simulink model "rfi_blk_v6_casper"  (Simulink 7.1)
settings: Solver=ode45  StartTime=0.0  StopTime=10.0  RelTol=1e-3
undefined symbols (not defined in the file): a, b
libraries: xbsIndex_r4 x13084, xps_library x4
(part 1 of 51)
settings Model: Creator=kaushal  LastModifiedBy=kaushal

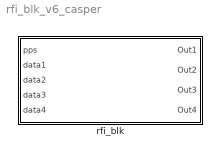
== system rfi_blk_v6_casper ==
  rfi_blk : Subsystem

== system rfi_blk_v6_casper/rfi_blk ==
  note: Median Absolute Deviation(MAD)
      |  Compuation Block
      | Computes median and MAD on 1024 bytes
      | of data for contiguous windows
      | Uses 1pps signal (active high - 1 clk) to restart
      | the computation
  note: RFI detection and filtering block
  note: RFI detection and filtering block
  note: RFI detection and filtering block
  note: RFI detection and filtering block
  note: 'n' value in median+/-n*sigma
  note: 0: Bypass
      | 1: Replace by constant value
      | 2: Replace by median value
      | 3: Replace with Digital Noise
  note: This design is divided into two blocks - MAD computation block and RFI detection and Filtering blocks
      | In the current version four RFI detection and filtering blocks are provided to cater to the four lanes from the ADC
  note: Replacement value for option 1 of bypass_mux
  pps : Inport  Orientation=up
  data1 : Inport  Port=2
  data2 : Inport  Port=3
  data3 : Inport  Port=4
  data4 : Inport  Port=5
  Constant1 : Constant  Value=10  OutDataType=sfix(16)  OutScaling=2^0
  Constant2 : Constant  Value=0  OutDataType=sfix(16)  OutScaling=2^0
  Constant3 : Constant  Value=3  OutDataType=sfix(16)  OutScaling=2^0
  Register{1,7..9} (4 alike) : xbsIndex_r4/Register  init=0  rst=off  en=off  dbl_ovrd=off  block_type=register
      settings: block_version=11.4
  Register{2..4,6} (4 alike) : xbsIndex_r4/Register  Orientation=up  init=0  rst=off  en=off  dbl_ovrd=off  block_type=register
      settings: block_version=11.4
  Reinterpret{9..12} (4 alike) : xbsIndex_r4/Reinterpret  force_arith_type=on  arith_type="Signed  (2's comp)"  force_bin_pt=on  bin_pt=0  block_type=reinterpret
      settings: infoedit="Changes signal type without altering the binary representation.   You can changed the signal between signed and unsigned, and relocate the binary point.<br><br>Hardware notes: In hardware this block costs nothing.<br><br>Example:  Suppose the input is 6 bits wide, signed, with 2 fractional bits, and the output is forced to unsigned with 0 fractional bits.  Then an input of -2.0 (1110.00 in binary 2's complement) becomes an output of 56 (111000 in binary)."
  Slice : xbsIndex_r4/Slice  nbits=8  boolean_output=off  mode=Lower Bit Location + Width  bit1=0  base1=MSB of Input  bit0=0  base0=LSB of Input  dbl_ovrd=off  block_type=slice
  Slice1 : xbsIndex_r4/Slice  nbits=8  boolean_output=off  mode=Lower Bit Location + Width  bit1=0  base1=MSB of Input  bit0=0  base0=LSB of Input  dbl_ovrd=off  block_type=slice
  Slice2 : xbsIndex_r4/Slice  nbits=2  boolean_output=off  mode=Lower Bit Location + Width  bit1=0  base1=MSB of Input  bit0=0  base0=LSB of Input  dbl_ovrd=off  block_type=slice
  bypass_mux : xps_library/software register  Tag=xps:sw_reg  SystemSampleTime=-1  io_dir=From Processor  arith_type=Unsigned  bitwidth=32  bin_pt=0  sample_period=1  gw_name=two_adc_rfi_v6_rfi_blk_bypass_mux_user_data_out
  cr1 : Subsystem
  cr2 : Subsystem
  cr4 : Subsystem
  cr5 : Subsystem
  mad_comp : Subsystem
  replacement : xps_library/software register  Tag=xps:sw_reg  SystemSampleTime=-1  io_dir=From Processor  arith_type=Unsigned  bitwidth=32  bin_pt=0  sample_period=1  gw_name=two_adc_rfi_v6_rfi_blk_replacement_user_data_out
  thres_fact : xps_library/software register  Tag=xps:sw_reg  SystemSampleTime=-1  io_dir=From Processor  arith_type=Unsigned  bitwidth=32  bin_pt=0  sample_period=1  gw_name=two_adc_rfi_v6_rfi_blk_thres_fact_user_data_out
  Out1 : Outport
  Out2 : Outport  Port=2
  Out3 : Outport  Port=3
  Out4 : Outport  Port=4
  wires:
  cr1/1(filter_o) -> Register1/1
  data2/1 -> Register7/1
  [med_valid] -> cr4/4(med_valid)
  [mad_valid] -> cr4/3(mad_valid)
  [mad] -> cr4/2(mad)
  [median] -> cr4/1(median)
  mad_comp/1(med_valid) -> [med_valid]
  mad_comp/2(median) -> [median]
  mad_comp/3(mad_valid) -> [mad_valid]
  mad_comp/4(mad) -> [mad]
  [med_valid] -> cr1/4(med_valid)
  [mad_valid] -> cr1/3(mad_valid)
  [mad] -> cr1/2(mad)
  [median] -> cr1/1(median)
  cr4/1(filter_o) -> Register2/1
  data3/1 -> Register8/1
  [med_valid] -> cr2/4(med_valid)
  [mad_valid] -> cr2/3(mad_valid)
  [mad] -> cr2/2(mad)
  [median] -> cr2/1(median)
  cr2/1(filter_o) -> Register3/1
  data4/1 -> Register9/1
  [med_valid] -> cr5/4(med_valid)
  [mad_valid] -> cr5/3(mad_valid)
  [mad] -> cr5/2(mad)
  [median] -> cr5/1(median)
  cr5/1(filter_o) -> Register4/1
  Reinterpret9/1 -> cr1/5(data_i)
  Reinterpret10/1 -> cr4/5(data_i)
  Reinterpret11/1 -> cr2/5(data_i)
  Reinterpret12/1 -> cr5/5(data_i)
  Register1/1 -> Out1/1
  Register2/1 -> Out2/1
  Register3/1 -> Out3/1
  Register4/1 -> Out4/1
  Register7/1 -> Reinterpret10/1
  Register8/1 -> Reinterpret11/1
  Register9/1 -> Reinterpret12/1
  pps/1 -> mad_comp/1(pps)
  data1/1 -> Register6/1
  thres_fact/1 -> Slice/1
  Constant1/1 -> thres_fact/1
  replacement/1 -> Slice1/1
  Constant2/1 -> replacement/1
  bypass_mux/1 -> Slice2/1
  Constant3/1 -> bypass_mux/1
  [thres] -> cr1/6(thr)
  [rep] -> cr1/7(rep)
  [bymux] -> cr1/8(bymux)
  Slice/1 -> [thres]
  Slice1/1 -> [rep]
  Slice2/1 -> [bymux]
  [thres] -> cr4/6(thr)
  [rep] -> cr4/7(rep)
  [bymux] -> cr4/8(bymux)
  [thres] -> cr2/6(thr)
  [rep] -> cr2/7(rep)
  [bymux] -> cr2/8(bymux)
  [thres] -> cr5/6(thr)
  [rep] -> cr5/7(rep)
  [bymux] -> cr5/8(bymux)
  Register6/1 -> mad_comp/2(data), Reinterpret9/1

== system rfi_blk_v6_casper/rfi_blk/cr1 ==
  note: Detection and Filtering (Clipping and Replacement) block
  median : Inport
  mad : Inport  Port=2
  mad_valid : Inport  Port=3
  med_valid : Inport  Port=4
  data_i : Inport  Port=5
  thr : Inport  Port=6
  rep : Inport  Port=7
  bymux : Inport  Port=8
  cr : Subsystem
  filter_o : Outport
  detect_o : Outport  Port=2
  data_o : Outport  Port=3
  wires:
  median/1 -> cr/1(median)
  cr/1(filter_o) -> filter_o/1
  mad/1 -> cr/2(mad1)
  mad_valid/1 -> cr/3(mad_valid)
  med_valid/1 -> cr/4(med_valid)
  cr/2(detect_o) -> detect_o/1
  data_i/1 -> cr/5(data_i)
  cr/3(data_o) -> data_o/1
  thr/1 -> cr/6(thres_fact)
  rep/1 -> cr/7(repl_val)
  bymux/1 -> cr/8(byp_mux)

== system rfi_blk_v6_casper/rfi_blk/cr2 ==
  note: Detection and Filtering (Clipping and Replacement) block
  median : Inport
  mad : Inport  Port=2
  mad_valid : Inport  Port=3
  med_valid : Inport  Port=4
  data_i : Inport  Port=5
  thr : Inport  Port=6
  rep : Inport  Port=7
  bymux : Inport  Port=8
  cr : Subsystem
  filter_o : Outport
  detect_o : Outport  Port=2
  data_o : Outport  Port=3
  wires:
  median/1 -> cr/1(median)
  cr/1(filter_o) -> filter_o/1
  mad/1 -> cr/2(mad1)
  mad_valid/1 -> cr/3(mad_valid)
  med_valid/1 -> cr/4(med_valid)
  cr/2(detect_o) -> detect_o/1
  data_i/1 -> cr/5(data_i)
  cr/3(data_o) -> data_o/1
  thr/1 -> cr/6(thres_fact)
  rep/1 -> cr/7(repl_val)
  bymux/1 -> cr/8(byp_mux)

== system rfi_blk_v6_casper/rfi_blk/cr4 ==
  note: Detection and Filtering (Clipping and Replacement) block
  median : Inport
  mad : Inport  Port=2
  mad_valid : Inport  Port=3
  med_valid : Inport  Port=4
  data_i : Inport  Port=5
  thr : Inport  Port=6
  rep : Inport  Port=7
  bymux : Inport  Port=8
  cr : Subsystem
  filter_o : Outport
  detect_o : Outport  Port=2
  data_o : Outport  Port=3
  wires:
  median/1 -> cr/1(median)
  cr/1(filter_o) -> filter_o/1
  mad/1 -> cr/2(mad1)
  mad_valid/1 -> cr/3(mad_valid)
  med_valid/1 -> cr/4(med_valid)
  cr/2(detect_o) -> detect_o/1
  data_i/1 -> cr/5(data_i)
  cr/3(data_o) -> data_o/1
  thr/1 -> cr/6(thres_fact)
  rep/1 -> cr/7(repl_val)
  bymux/1 -> cr/8(byp_mux)

== system rfi_blk_v6_casper/rfi_blk/cr5 ==
  note: Detection and Filtering (Clipping and Replacement) block
  median : Inport
  mad : Inport  Port=2
  mad_valid : Inport  Port=3
  med_valid : Inport  Port=4
  data_i : Inport  Port=5
  thr : Inport  Port=6
  rep : Inport  Port=7
  bymux : Inport  Port=8
  cr : Subsystem
  filter_o : Outport
  detect_o : Outport  Port=2
  data_o : Outport  Port=3
  wires:
  median/1 -> cr/1(median)
  cr/1(filter_o) -> filter_o/1
  mad/1 -> cr/2(mad1)
  mad_valid/1 -> cr/3(mad_valid)
  med_valid/1 -> cr/4(med_valid)
  cr/2(detect_o) -> detect_o/1
  data_i/1 -> cr/5(data_i)
  cr/3(data_o) -> data_o/1
  thr/1 -> cr/6(thres_fact)
  rep/1 -> cr/7(repl_val)
  bymux/1 -> cr/8(byp_mux)

== system rfi_blk_v6_casper/rfi_blk/mad_comp ==
  note: 0: Select pre-stored values from memory
      | 1: Input data
  note: First Median Computation
  note: Subtraction and Absolute Value Conversion
  note: Second Median Computation
  pps : Inport
  data : Inport  Port=2
  Constant1 : Constant  Value=0  OutDataType=sfix(16)  OutScaling=2^0
  Counter1 : xbsIndex_r4/Counter  cnt_type=Free Running  cnt_to=2048  operation=Up  start_count=0  cnt_by_val=1  arith_type=Unsigned  n_bits=13  bin_pt=0  load_pin=off  rst=off  en=off  explicit_period=on  period=1  dbl_ovrd=off  use_behavioral_HDL=off  implementation=Fabric  use_rpm=off  block_type=counter
  Delay2 : xbsIndex_r4/Delay  en=off  latency=3  dbl_ovrd=off  reg_retiming=off  block_type=delay
  Delay3 : xbsIndex_r4/Delay  Orientation=down  en=off  latency=1027+3  dbl_ovrd=off  reg_retiming=off  block_type=delay
  Delay4 : xbsIndex_r4/Delay  Orientation=down  en=off  latency=1027  dbl_ovrd=off  reg_retiming=off  block_type=delay
  Median1 : Subsystem
  Median2 : Subsystem
  Mux2 : xbsIndex_r4/Mux  inputs=2  en=off  latency=0  precision=User Defined  arith_type="Signed  (2's comp)"  n_bits=8  bin_pt=0  quantization=Truncate  overflow=Wrap  dbl_ovrd=off  block_type=mux
  ROM : xbsIndex_r4/ROM  depth=8192  initVector=0  distributed_mem=Block RAM  rst=off  init_reg=0  en=off  latency=1  arith_type="Signed  (2's comp)"  n_bits=8  bin_pt=0  dbl_ovrd=off  optimize=Area  use_rpm=on  block_type=sprom
      settings: block_version=10.1.3
  Reinterpret9 : xbsIndex_r4/Reinterpret  force_arith_type=on  arith_type="Signed  (2's comp)"  force_bin_pt=on  bin_pt=0  block_type=reinterpret
      settings: infoedit="Changes signal type without altering the binary representation.   You can changed the signal between signed and unsigned, and relocate the binary point.<br><br>Hardware notes: In hardware this block costs nothing.<br><br>Example:  Suppose the input is 6 bits wide, signed, with 2 fractional bits, and the output is forced to unsigned with 0 fractional bits.  Then an input of -2.0 (1110.00 in binary 2's complement) becomes an output of 56 (111000 in binary)."
  Scope3 : Scope  NumInputPorts=5
      settings: YMin=-1~5.7~-1~-1~-1  YMax=1~6.3~1~1~1  SaveName=ScopeData19  MaxDataPoints=30000
  Scope4 : Scope  NumInputPorts=5
      settings: YMin=81.7~-1~0.95~0.95~-1  YMax=90.3~1~1.05~1.05~1  SaveName=ScopeData22  MaxDataPoints=30000
  Slice : xbsIndex_r4/Slice  nbits=8  boolean_output=on  mode=Lower Bit Location + Width  bit1=0  base1=MSB of Input  bit0=0  base0=LSB of Input  dbl_ovrd=off  block_type=slice
  block_ram : Subsystem
  input_mux : xps_library/software register  Tag=xps:sw_reg  SystemSampleTime=-1  io_dir=From Processor  arith_type=Unsigned  bitwidth=32  bin_pt=0  sample_period=1  gw_name=two_adc_rfi_v6_rfi_blk_mad_comp_input_mux_user_data_out
  sub_abs_conv : Subsystem
  sync_control : Subsystem
  med_valid : Outport
  median : Outport  Port=2
  mad_valid : Outport  Port=3
  mad : Outport  Port=4
  wires:
  Counter1/1 -> ROM/1
  Median2/1(med_out) -> Delay4/1, sub_abs_conv/2(In2)
  sub_abs_conv/1(Out1) -> Median1/1(data_in)
  Median1/1(med_out) -> Scope4/1, mad/1
  Delay2/1 -> Median1/2(acc_reset), Scope4/3
  sync_control/1(Out1) -> Median2/2(acc_reset), Delay2/1
  block_ram/2(Out2) -> sub_abs_conv/1(In1)
  Median2/3(med_valid1) -> Delay3/1
  Median1/4(mad_valid) -> Scope4/4, mad_valid/1
  Median1/2(med_valid) -> Scope4/5
  Delay4/1 -> Scope3/2, median/1
  Delay3/1 -> Scope3/1, med_valid/1
  pps/1 -> sync_control/1(pps)
  Mux2/1 -> Median2/1(data_in), block_ram/1(data)
  data/1 -> Reinterpret9/1
  ROM/1 -> Mux2/3
  input_mux/1 -> Slice/1
  Constant1/1 -> input_mux/1
  Slice/1 -> Mux2/1
  Reinterpret9/1 -> Mux2/2

== system rfi_blk_v6_casper/rfi_blk/cr1/cr ==
  note: Detection Threshold Computation
  note: RFI Filtering (Clipping / Replacement)
  median : Inport
  mad1 : Inport  Port=2
  mad_valid : Inport  Port=3
  med_valid : Inport  Port=4
  data_i : Inport  Port=5
  thres_fact : Inport  Port=6
  repl_val : Inport  Port=7
  byp_mux : Inport  Port=8
  Delay1 : xbsIndex_r4/Delay  en=off  latency=10  dbl_ovrd=off  reg_retiming=off  block_type=delay
  Delay5 : xbsIndex_r4/Delay  en=off  latency=1027+1024  dbl_ovrd=off  reg_retiming=off  block_type=delay
  Mux1 : xbsIndex_r4/Mux  inputs=2  en=off  latency=0  precision=User Defined  arith_type="Signed  (2's comp)"  n_bits=8  bin_pt=0  quantization=Truncate  overflow=Wrap  dbl_ovrd=off  block_type=mux
  Mux2 : xbsIndex_r4/Mux  inputs=2  en=off  latency=0  precision=User Defined  arith_type=Unsigned  n_bits=8  bin_pt=0  quantization=Truncate  overflow=Wrap  dbl_ovrd=off  block_type=mux
  Register : xbsIndex_r4/Register  init=0  rst=off  en=off  dbl_ovrd=off  block_type=register
      settings: block_version=11.4
  Register1 : xbsIndex_r4/Register  init=0  rst=off  en=off  dbl_ovrd=off  block_type=register
      settings: block_version=11.4
  Scope1 : Scope  NumInputPorts=4
      settings: YMin=-1~-1~-150~-1  YMax=1~1~150~1  MaxDataPoints=30000
  Scope2 : Scope  NumInputPorts=4
      settings: YMin=-1~0.95~-125~-1  YMax=1~1.05~150~1  MaxDataPoints=30000
  clip_repl : Subsystem
  thres_calc : Subsystem
  filter_o : Outport
  detect_o : Outport  Port=2
  data_o : Outport  Port=3
  wires:
  clip_repl/1(filter_o) -> Scope2/1, filter_o/1
  Delay5/1 -> Delay1/1
  clip_repl/3(input_data) -> data_o/1, Scope2/3
  clip_repl/2(detect_o) -> Scope2/2, detect_o/1
  thres_calc/3(sigma_mad_o) -> clip_repl/5(sigma_mad)
  Register1/1 -> thres_calc/1(mad), Mux2/2
  Mux2/1 -> Register1/1
  Register/1 -> Mux1/2, thres_calc/2(median), clip_repl/4(median)
  Mux1/1 -> Register/1
  Delay1/1 -> clip_repl/3(input), Scope1/3
  thres_calc/2(n_th) -> clip_repl/2(n_thres), Scope1/2
  thres_calc/1(p_th) -> clip_repl/1(p_thres), Scope1/1
  mad1/1 -> Mux2/3
  mad_valid/1 -> Mux2/1
  median/1 -> Mux1/3
  med_valid/1 -> Mux1/1
  data_i/1 -> Delay5/1
  thres_fact/1 -> thres_calc/3(thres_fact)
  repl_val/1 -> clip_repl/6(repl_val)
  byp_mux/1 -> clip_repl/7(bypass_mux)

== system rfi_blk_v6_casper/rfi_blk/cr2/cr ==
  note: Detection Threshold Computation
  note: RFI Filtering (Clipping / Replacement)
  median : Inport
  mad1 : Inport  Port=2
  mad_valid : Inport  Port=3
  med_valid : Inport  Port=4
  data_i : Inport  Port=5
  thres_fact : Inport  Port=6
  repl_val : Inport  Port=7
  byp_mux : Inport  Port=8
  Delay1 : xbsIndex_r4/Delay  en=off  latency=10  dbl_ovrd=off  reg_retiming=off  block_type=delay
  Delay5 : xbsIndex_r4/Delay  en=off  latency=1027+1024  dbl_ovrd=off  reg_retiming=off  block_type=delay
  Mux1 : xbsIndex_r4/Mux  inputs=2  en=off  latency=0  precision=User Defined  arith_type="Signed  (2's comp)"  n_bits=8  bin_pt=0  quantization=Truncate  overflow=Wrap  dbl_ovrd=off  block_type=mux
  Mux2 : xbsIndex_r4/Mux  inputs=2  en=off  latency=0  precision=User Defined  arith_type=Unsigned  n_bits=8  bin_pt=0  quantization=Truncate  overflow=Wrap  dbl_ovrd=off  block_type=mux
  Register : xbsIndex_r4/Register  init=0  rst=off  en=off  dbl_ovrd=off  block_type=register
      settings: block_version=11.4
  Register1 : xbsIndex_r4/Register  init=0  rst=off  en=off  dbl_ovrd=off  block_type=register
      settings: block_version=11.4
  Scope1 : Scope  NumInputPorts=4
      settings: YMin=-1~-1~-150~-1  YMax=1~1~150~1  MaxDataPoints=30000
  Scope2 : Scope  NumInputPorts=4
      settings: YMin=-1~0.95~-125~-1  YMax=1~1.05~150~1  MaxDataPoints=30000
  clip_repl : Subsystem
  thres_calc : Subsystem
  filter_o : Outport
  detect_o : Outport  Port=2
  data_o : Outport  Port=3
  wires:
  clip_repl/1(filter_o) -> Scope2/1, filter_o/1
  Delay5/1 -> Delay1/1
  clip_repl/3(input_data) -> data_o/1, Scope2/3
  clip_repl/2(detect_o) -> Scope2/2, detect_o/1
  thres_calc/3(sigma_mad_o) -> clip_repl/5(sigma_mad)
  Register1/1 -> thres_calc/1(mad), Mux2/2
  Mux2/1 -> Register1/1
  Register/1 -> Mux1/2, thres_calc/2(median), clip_repl/4(median)
  Mux1/1 -> Register/1
  Delay1/1 -> clip_repl/3(input), Scope1/3
  thres_calc/2(n_th) -> clip_repl/2(n_thres), Scope1/2
  thres_calc/1(p_th) -> clip_repl/1(p_thres), Scope1/1
  mad1/1 -> Mux2/3
  mad_valid/1 -> Mux2/1
  median/1 -> Mux1/3
  med_valid/1 -> Mux1/1
  data_i/1 -> Delay5/1
  thres_fact/1 -> thres_calc/3(thres_fact)
  repl_val/1 -> clip_repl/6(repl_val)
  byp_mux/1 -> clip_repl/7(bypass_mux)

== system rfi_blk_v6_casper/rfi_blk/cr4/cr ==
  note: Detection Threshold Computation
  note: RFI Filtering (Clipping / Replacement)
  median : Inport
  mad1 : Inport  Port=2
  mad_valid : Inport  Port=3
  med_valid : Inport  Port=4
  data_i : Inport  Port=5
  thres_fact : Inport  Port=6
  repl_val : Inport  Port=7
  byp_mux : Inport  Port=8
  Delay1 : xbsIndex_r4/Delay  en=off  latency=10  dbl_ovrd=off  reg_retiming=off  block_type=delay
  Delay5 : xbsIndex_r4/Delay  en=off  latency=1027+1024  dbl_ovrd=off  reg_retiming=off  block_type=delay
  Mux1 : xbsIndex_r4/Mux  inputs=2  en=off  latency=0  precision=User Defined  arith_type="Signed  (2's comp)"  n_bits=8  bin_pt=0  quantization=Truncate  overflow=Wrap  dbl_ovrd=off  block_type=mux
  Mux2 : xbsIndex_r4/Mux  inputs=2  en=off  latency=0  precision=User Defined  arith_type=Unsigned  n_bits=8  bin_pt=0  quantization=Truncate  overflow=Wrap  dbl_ovrd=off  block_type=mux
  Register : xbsIndex_r4/Register  init=0  rst=off  en=off  dbl_ovrd=off  block_type=register
      settings: block_version=11.4
  Register1 : xbsIndex_r4/Register  init=0  rst=off  en=off  dbl_ovrd=off  block_type=register
      settings: block_version=11.4
  Scope1 : Scope  NumInputPorts=4
      settings: YMin=-1~-1~-150~-1  YMax=1~1~150~1  MaxDataPoints=30000
  Scope2 : Scope  NumInputPorts=4
      settings: YMin=-1~0.95~-125~-1  YMax=1~1.05~150~1  MaxDataPoints=30000
  clip_repl : Subsystem
  thres_calc : Subsystem
  filter_o : Outport
  detect_o : Outport  Port=2
  data_o : Outport  Port=3
  wires:
  clip_repl/1(filter_o) -> Scope2/1, filter_o/1
  Delay5/1 -> Delay1/1
  clip_repl/3(input_data) -> data_o/1, Scope2/3
  clip_repl/2(detect_o) -> Scope2/2, detect_o/1
  thres_calc/3(sigma_mad_o) -> clip_repl/5(sigma_mad)
  Register1/1 -> thres_calc/1(mad), Mux2/2
  Mux2/1 -> Register1/1
  Register/1 -> Mux1/2, thres_calc/2(median), clip_repl/4(median)
  Mux1/1 -> Register/1
  Delay1/1 -> clip_repl/3(input), Scope1/3
  thres_calc/2(n_th) -> clip_repl/2(n_thres), Scope1/2
  thres_calc/1(p_th) -> clip_repl/1(p_thres), Scope1/1
  mad1/1 -> Mux2/3
  mad_valid/1 -> Mux2/1
  median/1 -> Mux1/3
  med_valid/1 -> Mux1/1
  data_i/1 -> Delay5/1
  thres_fact/1 -> thres_calc/3(thres_fact)
  repl_val/1 -> clip_repl/6(repl_val)
  byp_mux/1 -> clip_repl/7(bypass_mux)

== system rfi_blk_v6_casper/rfi_blk/cr5/cr ==
  note: Detection Threshold Computation
  note: RFI Filtering (Clipping / Replacement)
  median : Inport
  mad1 : Inport  Port=2
  mad_valid : Inport  Port=3
  med_valid : Inport  Port=4
  data_i : Inport  Port=5
  thres_fact : Inport  Port=6
  repl_val : Inport  Port=7
  byp_mux : Inport  Port=8
  Delay1 : xbsIndex_r4/Delay  en=off  latency=10  dbl_ovrd=off  reg_retiming=off  block_type=delay
  Delay5 : xbsIndex_r4/Delay  en=off  latency=1027+1024  dbl_ovrd=off  reg_retiming=off  block_type=delay
  Mux1 : xbsIndex_r4/Mux  inputs=2  en=off  latency=0  precision=User Defined  arith_type="Signed  (2's comp)"  n_bits=8  bin_pt=0  quantization=Truncate  overflow=Wrap  dbl_ovrd=off  block_type=mux
  Mux2 : xbsIndex_r4/Mux  inputs=2  en=off  latency=0  precision=User Defined  arith_type=Unsigned  n_bits=8  bin_pt=0  quantization=Truncate  overflow=Wrap  dbl_ovrd=off  block_type=mux
  Register : xbsIndex_r4/Register  init=0  rst=off  en=off  dbl_ovrd=off  block_type=register
      settings: block_version=11.4
  Register1 : xbsIndex_r4/Register  init=0  rst=off  en=off  dbl_ovrd=off  block_type=register
      settings: block_version=11.4
  Scope1 : Scope  NumInputPorts=4
      settings: YMin=-1~-1~-150~-1  YMax=1~1~150~1  MaxDataPoints=30000
  Scope2 : Scope  NumInputPorts=4
      settings: YMin=-1~0.95~-125~-1  YMax=1~1.05~150~1  MaxDataPoints=30000
  clip_repl : Subsystem
  thres_calc : Subsystem
  filter_o : Outport
  detect_o : Outport  Port=2
  data_o : Outport  Port=3
  wires:
  clip_repl/1(filter_o) -> Scope2/1, filter_o/1
  Delay5/1 -> Delay1/1
  clip_repl/3(input_data) -> data_o/1, Scope2/3
  clip_repl/2(detect_o) -> Scope2/2, detect_o/1
  thres_calc/3(sigma_mad_o) -> clip_repl/5(sigma_mad)
  Register1/1 -> thres_calc/1(mad), Mux2/2
  Mux2/1 -> Register1/1
  Register/1 -> Mux1/2, thres_calc/2(median), clip_repl/4(median)
  Mux1/1 -> Register/1
  Delay1/1 -> clip_repl/3(input), Scope1/3
  thres_calc/2(n_th) -> clip_repl/2(n_thres), Scope1/2
  thres_calc/1(p_th) -> clip_repl/1(p_thres), Scope1/1
  mad1/1 -> Mux2/3
  mad_valid/1 -> Mux2/1
  median/1 -> Mux1/3
  med_valid/1 -> Mux1/1
  data_i/1 -> Delay5/1
  thres_fact/1 -> thres_calc/3(thres_fact)
  repl_val/1 -> clip_repl/6(repl_val)
  byp_mux/1 -> clip_repl/7(bypass_mux)

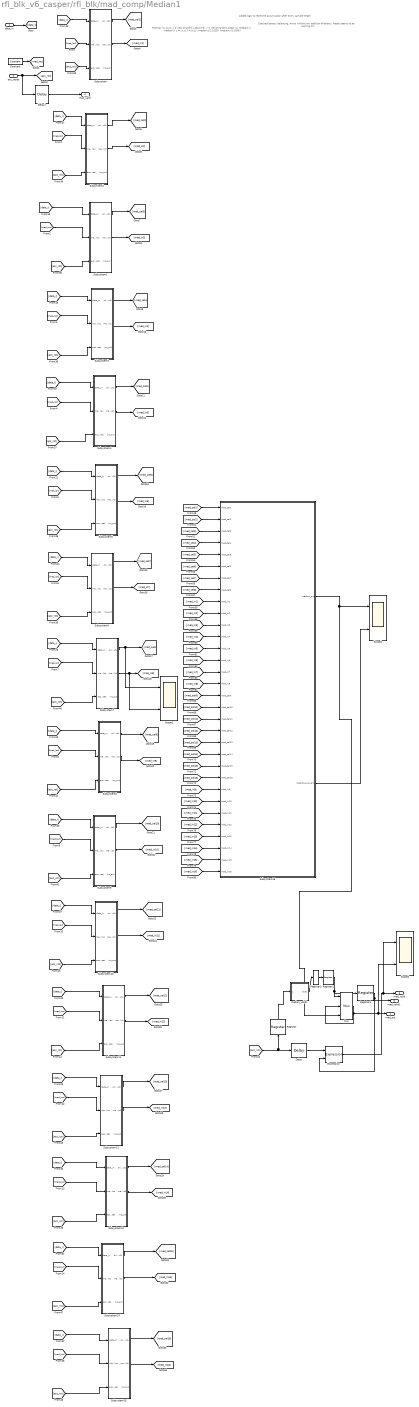
== system rfi_blk_v6_casper/rfi_blk/mad_comp/Median1 ==
  note: Testing:
      | f = [t;x]';
      | x = ceil(4*randn(1,1024)+5);
      | y = ceil(2*randn(1,1024)-4);
      | median(x)
      | median(y)
      | z = [x,y];
      | f = [t;z]';
      | median(z(1:1025))
      | median(z(1:1030))
  note: Added logic to reset the accumulator after every sample length
  note: Checked latency balancing, works without any addition of latency. Reset seems to be working OK.
  data_in : Inport  OutDataType=sfix(16)  OutScaling=2^0
  acc_reset : Inport  Port=2  OutDataType=sfix(16)  OutScaling=2^0
  Constant : xbsIndex_r4/Constant  arith_type=Unsigned  const=512  n_bits=10  bin_pt=0  explicit_period=on  period=1  equ=P=C  opselect=C  inp2=PCIN>>17  opr=+  inp1=P  carry=CIN  dbl_ovrd=off  block_type=constant
  Delay : xbsIndex_r4/Delay  en=off  latency=4  dbl_ovrd=off  reg_retiming=off  block_type=delay
  Delay1 : xbsIndex_r4/Delay  en=off  latency=1029  dbl_ovrd=off  reg_retiming=off  block_type=delay
  Expression : xbsIndex_r4/Expression  expression=a & b  align_bp=on  en=off  latency=0  precision=Full  arith_type=Unsigned  n_bits=16  bin_pt=0  dbl_ovrd=off  block_type=expr
      settings: block_version=10.1.3
  Mux : xbsIndex_r4/Mux  inputs=2  en=off  latency=1  precision=Full  arith_type=Unsigned  n_bits=16  bin_pt=14  quantization=Truncate  overflow=Wrap  dbl_ovrd=off  block_type=mux
  Register : xbsIndex_r4/Register  Orientation=up  init=0  rst=off  en=off  dbl_ovrd=off  block_type=register
  Register1 : xbsIndex_r4/Register  init=0  rst=off  en=off  dbl_ovrd=off  block_type=register
  Register2 : xbsIndex_r4/Register  init=0  rst=off  en=off  dbl_ovrd=off  block_type=register
  Register3 : xbsIndex_r4/Register  init=0  rst=off  en=off  dbl_ovrd=off  block_type=register
  Scope1 : Scope  NumInputPorts=2
      settings: YMin=-5~-5  YMax=5~5  SaveName=ScopeData13
  Scope2 : Scope  NumInputPorts=2
      settings: YMin=-5~-5  YMax=5~5  SaveName=ScopeData18
  Scope3 : Scope  NumInputPorts=2
      settings: YMin=-5~-5  YMax=5~5  SaveName=ScopeData6
  Subsystem : Subsystem  mask{bin_val1=0, bin_val2=1, bin_val3=2, bin_val4=3, bin_val5=4, bin_val6=5, bin_val7=6, bin_val8=7, bin_val9=8, bin_val10=9, bin_val11=10, bin_val12=11, bin_val13=12, bin_val14=13, bin_val15=14, bin_val16=15}
  Subsystem1 : Subsystem  mask{bin_val1=32, bin_val2=33, bin_val3=34, bin_val4=35, bin_val5=36, bin_val6=37, bin_val7=38, bin_val8=39, bin_val9=40, bin_val10=41, bin_val11=42, bin_val12=43, bin_val13=44, bin_val14=45, bin_val15=46, bin_val16=47}
  Subsystem10 : Subsystem  mask{bin_val1=160, bin_val2=161, bin_val3=162, bin_val4=163, bin_val5=164, bin_val6=165, bin_val7=166, bin_val8=167, bin_val9=168, bin_val10=169, bin_val11=170, bin_val12=171, bin_val13=172, bin_val14=173, bin_val15=174, bin_val16=175}
  Subsystem11 : Subsystem  mask{bin_val1=176, bin_val2=177, bin_val3=178, bin_val4=179, bin_val5=180, bin_val6=181, bin_val7=182, bin_val8=183, bin_val9=184, bin_val10=185, bin_val11=186, bin_val12=187, bin_val13=188, bin_val14=189, bin_val15=190, bin_val16=191}
  Subsystem12 : Subsystem  mask{bin_val1=192, bin_val2=193, bin_val3=194, bin_val4=195, bin_val5=196, bin_val6=197, bin_val7=198, bin_val8=199, bin_val9=200, bin_val10=201, bin_val11=202, bin_val12=203, bin_val13=204, bin_val14=205, bin_val15=206, bin_val16=207}
  Subsystem13 : Subsystem  mask{bin_val1=208, bin_val2=209, bin_val3=210, bin_val4=211, bin_val5=212, bin_val6=213, bin_val7=214, bin_val8=215, bin_val9=216, bin_val10=217, bin_val11=218, bin_val12=219, bin_val13=220, bin_val14=221, bin_val15=222, bin_val16=223}
  Subsystem14 : Subsystem  mask{bin_val1=224, bin_val2=225, bin_val3=226, bin_val4=227, bin_val5=228, bin_val6=229, bin_val7=230, bin_val8=231, bin_val9=232, bin_val10=233, bin_val11=234, bin_val12=235, bin_val13=236, bin_val14=237, bin_val15=238, bin_val16=239}
  Subsystem15 : Subsystem  mask{bin_val1=240, bin_val2=241, bin_val3=242, bin_val4=243, bin_val5=244, bin_val6=245, bin_val7=246, bin_val8=247, bin_val9=248, bin_val10=249, bin_val11=250, bin_val12=251, bin_val13=252, bin_val14=253, bin_val15=254, bin_val16=255}
  Subsystem16 : Subsystem
  Subsystem2 : Subsystem  mask{bin_val1=16, bin_val2=17, bin_val3=18, bin_val4=19, bin_val5=20, bin_val6=21, bin_val7=22, bin_val8=23, bin_val9=24, bin_val10=25, bin_val11=26, bin_val12=27, bin_val13=28, bin_val14=29, bin_val15=30, bin_val16=31}
  Subsystem3 : Subsystem  mask{bin_val1=48, bin_val2=49, bin_val3=50, bin_val4=51, bin_val5=52, bin_val6=53, bin_val7=54, bin_val8=55, bin_val9=56, bin_val10=57, bin_val11=58, bin_val12=59, bin_val13=60, bin_val14=61, bin_val15=62, bin_val16=63}
  Subsystem4 : Subsystem  mask{bin_val1=64, bin_val2=65, bin_val3=66, bin_val4=67, bin_val5=68, bin_val6=69, bin_val7=70, bin_val8=71, bin_val9=72, bin_val10=73, bin_val11=74, bin_val12=75, bin_val13=76, bin_val14=77, bin_val15=78, bin_val16=79}
  Subsystem5 : Subsystem  mask{bin_val1=80, bin_val2=81, bin_val3=82, bin_val4=83, bin_val5=84, bin_val6=85, bin_val7=86, bin_val8=87, bin_val9=88, bin_val10=89, bin_val11=90, bin_val12=91, bin_val13=92, bin_val14=93, bin_val15=94, bin_val16=95}
  Subsystem6 : Subsystem  mask{bin_val1=96, bin_val2=97, bin_val3=98, bin_val4=99, bin_val5=100, bin_val6=101, bin_val7=102, bin_val8=103, bin_val9=104, bin_val10=105, bin_val11=106, bin_val12=107, bin_val13=108, bin_val14=109, bin_val15=110, bin_val16=111}
  Subsystem7 : Subsystem  mask{bin_val1=112, bin_val2=113, bin_val3=114, bin_val4=115, bin_val5=116, bin_val6=117, bin_val7=118, bin_val8=119, bin_val9=120, bin_val10=121, bin_val11=122, bin_val12=123, bin_val13=124, bin_val14=125, bin_val15=126, bin_val16=127}
  Subsystem8 : Subsystem  mask{bin_val1=128, bin_val2=129, bin_val3=130, bin_val4=131, bin_val5=132, bin_val6=133, bin_val7=134, bin_val8=135, bin_val9=136, bin_val10=137, bin_val11=138, bin_val12=139, bin_val13=140, bin_val14=141, bin_val15=142, bin_val16=143}
  Subsystem9 : Subsystem  mask{bin_val1=144, bin_val2=145, bin_val3=146, bin_val4=147, bin_val5=148, bin_val6=149, bin_val7=150, bin_val8=151, bin_val9=152, bin_val10=153, bin_val11=154, bin_val12=155, bin_val13=156, bin_val14=157, bin_val15=158, bin_val16=159}
  median_valid1 : Subsystem
  med_out : Outport
  med_valid : Outport  Port=2
  med_valid1 : Outport  Port=3
  mad_valid : Outport  Port=4
  wires:
  Register/1 -> median_valid1/1(reset_in)
  Mux/1 -> Mux/2, med_out/1, Scope2/2
  [med_loc] -> Subsystem/2(med_loc)
  [med_loc] -> Subsystem2/2(med_loc)
  [med_loc] -> Subsystem1/2(med_loc)
  [med_loc] -> Subsystem3/2(med_loc)
  [med_loc] -> Subsystem4/2(med_loc)
  [med_loc] -> Subsystem5/2(med_loc)
  [med_loc] -> Subsystem6/2(med_loc)
  [med_loc] -> Subsystem7/2(med_loc)
  [med_loc] -> Subsystem8/2(med_loc)
  [med_loc] -> Subsystem9/2(med_loc)
  [med_loc] -> Subsystem10/2(med_loc)
  [med_loc] -> Subsystem11/2(med_loc)
  [med_loc] -> Subsystem12/2(med_loc)
  [med_loc] -> Subsystem13/2(med_loc)
  [med_loc] -> Subsystem14/2(med_loc)
  [med_loc] -> Subsystem15/2(med_loc)
  [data_i] -> Subsystem/1(data_in)
  [data_i] -> Subsystem2/1(data_in)
  [data_i] -> Subsystem1/1(data_in)
  [data_i] -> Subsystem3/1(data_in)
  [data_i] -> Subsystem4/1(data_in)
  [data_i] -> Subsystem5/1(data_in)
  [data_i] -> Subsystem6/1(data_in)
  [data_i] -> Subsystem7/1(data_in)
  [data_i] -> Subsystem8/1(data_in)
  [data_i] -> Subsystem9/1(data_in)
  [data_i] -> Subsystem10/1(data_in)
  [data_i] -> Subsystem11/1(data_in)
  [data_i] -> Subsystem12/1(data_in)
  [data_i] -> Subsystem13/1(data_in)
  [data_i] -> Subsystem14/1(data_in)
  [data_i] -> Subsystem15/1(data_in)
  [acc_rst] -> Subsystem/3(acc_reset)
  [acc_rst] -> Subsystem2/3(acc_reset)
  [acc_rst] -> Subsystem1/3(acc_reset)
  [acc_rst] -> Subsystem3/3(acc_reset)
  [acc_rst] -> Subsystem4/3(acc_reset)
  [acc_rst] -> Subsystem5/3(acc_reset)
  [acc_rst] -> Subsystem6/3(acc_reset)
  [acc_rst] -> Subsystem7/3(acc_reset)
  [acc_rst] -> Subsystem8/3(acc_reset)
  [acc_rst] -> Subsystem9/3(acc_reset)
  [acc_rst] -> Subsystem10/3(acc_reset)
  [acc_rst] -> Subsystem11/3(acc_reset)
  [acc_rst] -> Subsystem12/3(acc_reset)
  [acc_rst] -> Subsystem13/3(acc_reset)
  [acc_rst] -> Subsystem14/3(acc_reset)
  [acc_rst] -> Subsystem15/3(acc_reset)
  data_in/1 -> [data_i]
  Constant/1 -> [med_loc]
  acc_reset/1 -> [acc_rst], Delay1/1
  Subsystem16/1(median_out) -> Mux/3, Scope3/1
  [acc_rst] -> Register/1, Delay/1
  Subsystem/1(priority_out1) -> [med_sel1]
  Subsystem/2(med_calc1) -> [med_in1]
  Subsystem2/1(priority_out1) -> [med_sel2]
  Subsystem2/2(med_calc1) -> [med_in2]
  Subsystem1/1(priority_out1) -> [med_sel3]
  Subsystem1/2(med_calc1) -> [med_in3]
  Subsystem3/1(priority_out1) -> [med_sel4]
  Subsystem3/2(med_calc1) -> [med_in4]
  Subsystem4/1(priority_out1) -> [med_sel5]
  Subsystem4/2(med_calc1) -> [med_in5]
  Subsystem5/1(priority_out1) -> [med_sel6]
  Subsystem5/2(med_calc1) -> [med_in6]
  Subsystem6/1(priority_out1) -> [med_sel7]
  Subsystem6/2(med_calc1) -> [med_in7]
  Subsystem7/1(priority_out1) -> [med_sel8], Scope1/1
  Subsystem7/2(med_calc1) -> [med_in8], Scope1/2
  Subsystem8/1(priority_out1) -> [med_sel9]
  Subsystem8/2(med_calc1) -> [med_in9]
  Subsystem9/1(priority_out1) -> [med_sel10]
  Subsystem9/2(med_calc1) -> [med_in10]
  Subsystem10/1(priority_out1) -> [med_sel11]
  Subsystem10/2(med_calc1) -> [med_in11]
  Subsystem11/1(priority_out1) -> [med_sel12]
  Subsystem11/2(med_calc1) -> [med_in12]
  Subsystem12/1(priority_out1) -> [med_sel13]
  Subsystem12/2(med_calc1) -> [med_in13]
  Subsystem13/1(priority_out1) -> [med_sel14]
  Subsystem13/2(med_calc1) -> [med_in14]
  Subsystem14/1(priority_out1) -> [med_sel15]
  Subsystem14/2(med_calc1) -> [med_in15]
  Subsystem15/1(priority_out1) -> [med_sel16]
  Subsystem15/2(med_calc1) -> [med_in16]
  [med_sel1] -> Subsystem16/1(med_sel1)
  [med_in1] -> Subsystem16/9(med_in1)
  [med_sel2] -> Subsystem16/2(med_sel2)
  [med_sel3] -> Subsystem16/3(med_sel3)
  [med_sel4] -> Subsystem16/4(med_sel4)
  [med_sel5] -> Subsystem16/5(med_sel5)
  [med_sel6] -> Subsystem16/6(med_sel6)
  [med_sel7] -> Subsystem16/7(med_sel7)
  [med_sel8] -> Subsystem16/8(med_sel8)
  [med_in2] -> Subsystem16/10(med_in2)
  [med_in3] -> Subsystem16/11(med_in3)
  [med_in4] -> Subsystem16/12(med_in4)
  [med_in5] -> Subsystem16/13(med_in5)
  [med_in6] -> Subsystem16/14(med_in6)
  [med_in7] -> Subsystem16/15(med_in7)
  [med_in8] -> Subsystem16/16(med_in8)
  [med_sel9] -> Subsystem16/17(med_sel9)
  [med_sel10] -> Subsystem16/18(med_sel10)
  [med_sel11] -> Subsystem16/19(med_sel11)
  [med_sel12] -> Subsystem16/20(med_sel12)
  [med_sel13] -> Subsystem16/21(med_sel13)
  [med_sel14] -> Subsystem16/22(med_sel14)
  [med_sel15] -> Subsystem16/23(med_sel15)
  [med_sel16] -> Subsystem16/24(med_sel16)
  [med_in9] -> Subsystem16/25(med_in9)
  [med_in10] -> Subsystem16/26(med_in10)
  [med_in11] -> Subsystem16/27(med_in11)
  [med_in12] -> Subsystem16/28(med_in12)
  [med_in13] -> Subsystem16/29(med_in13)
  [med_in14] -> Subsystem16/30(med_in14)
  [med_in15] -> Subsystem16/31(med_in15)
  [med_in16] -> Subsystem16/32(med_in16)
  Subsystem16/2(median_out_valid) -> Scope3/2
  Register2/1 -> Mux/1, Register1/1
  median_valid1/1(Out1) -> Register3/1
  Register3/1 -> Register2/1
  Delay/1 -> Expression/1
  Register1/1 -> Expression/2, med_valid1/1
  Expression/1 -> med_valid/1, Scope2/1
  Delay1/1 -> mad_valid/1

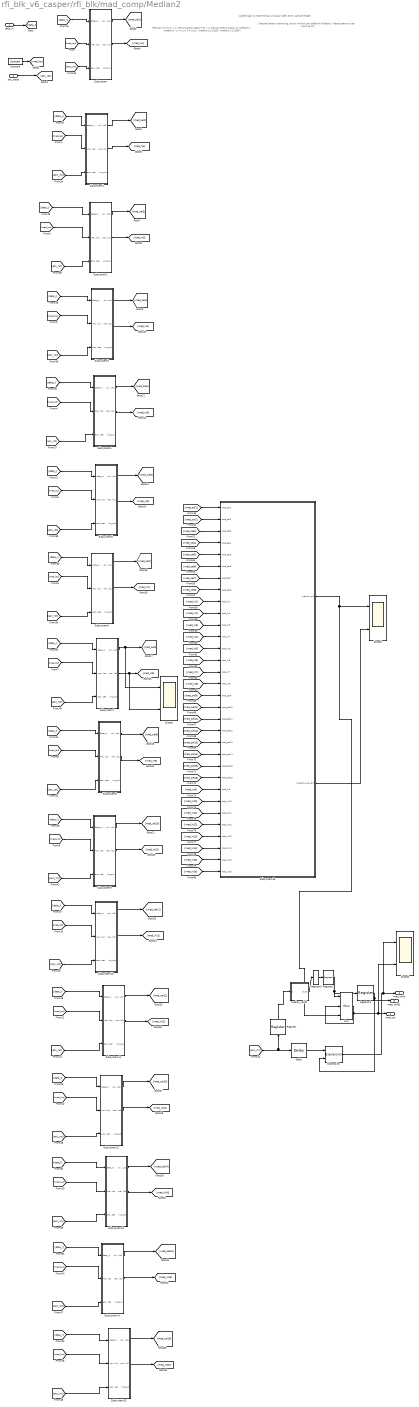
== system rfi_blk_v6_casper/rfi_blk/mad_comp/Median2 ==
  note: Testing:
      | f = [t;x]';
      | x = ceil(4*randn(1,1024)+5);
      | y = ceil(2*randn(1,1024)-4);
      | median(x)
      | median(y)
      | z = [x,y];
      | f = [t;z]';
      | median(z(1:1025))
      | median(z(1:1030))
  note: Added logic to reset the accumulator after every sample length
  note: Checked latency balancing, works without any addition of latency. Reset seems to be working OK.
  data_in : Inport  OutDataType=sfix(16)  OutScaling=2^0
  acc_reset : Inport  Port=2  OutDataType=sfix(16)  OutScaling=2^0
  Constant : xbsIndex_r4/Constant  arith_type=Unsigned  const=512  n_bits=10  bin_pt=0  explicit_period=on  period=1  equ=P=C  opselect=C  inp2=PCIN>>17  opr=+  inp1=P  carry=CIN  dbl_ovrd=off  block_type=constant
  Delay : xbsIndex_r4/Delay  en=off  latency=4  dbl_ovrd=off  reg_retiming=off  block_type=delay
  Expression : xbsIndex_r4/Expression  expression=a & b  align_bp=on  en=off  latency=0  precision=Full  arith_type=Unsigned  n_bits=16  bin_pt=0  dbl_ovrd=off  block_type=expr
      settings: block_version=10.1.3
  Mux : xbsIndex_r4/Mux  inputs=2  en=off  latency=1  precision=Full  arith_type=Unsigned  n_bits=16  bin_pt=14  quantization=Truncate  overflow=Wrap  dbl_ovrd=off  block_type=mux
  Register : xbsIndex_r4/Register  Orientation=up  init=0  rst=off  en=off  dbl_ovrd=off  block_type=register
  Register1 : xbsIndex_r4/Register  init=0  rst=off  en=off  dbl_ovrd=off  block_type=register
  Register2 : xbsIndex_r4/Register  init=0  rst=off  en=off  dbl_ovrd=off  block_type=register
  Register3 : xbsIndex_r4/Register  init=0  rst=off  en=off  dbl_ovrd=off  block_type=register
  Scope1 : Scope  NumInputPorts=2
      settings: YMin=-5~-5  YMax=5~5  SaveName=ScopeData13
  Scope2 : Scope  NumInputPorts=2
      settings: YMin=-5~-5  YMax=5~5  SaveName=ScopeData18
  Scope3 : Scope  NumInputPorts=2
      settings: YMin=-5~-5  YMax=5~5  SaveName=ScopeData6
  Subsystem : Subsystem  mask{bin_val1=-128, bin_val2=-127, bin_val3=-126, bin_val4=-125, bin_val5=-124, bin_val6=-123, bin_val7=-122, bin_val8=-121, bin_val9=-120, bin_val10=-119, bin_val11=-118, bin_val12=-117, bin_val13=-116, bin_val14=-115, bin_val15=-114, bin_val16=-113}
  Subsystem1 : Subsystem  mask{bin_val1=-96, bin_val2=-95, bin_val3=-94, bin_val4=-93, bin_val5=-92, bin_val6=-91, bin_val7=-90, bin_val8=-89, bin_val9=-88, bin_val10=-87, bin_val11=-86, bin_val12=-85, bin_val13=-84, bin_val14=-83, bin_val15=-82, bin_val16=-81}
  Subsystem10 : Subsystem  mask{bin_val1=32, bin_val2=33, bin_val3=34, bin_val4=35, bin_val5=36, bin_val6=37, bin_val7=38, bin_val8=39, bin_val9=40, bin_val10=41, bin_val11=42, bin_val12=43, bin_val13=44, bin_val14=45, bin_val15=46, bin_val16=47}
  Subsystem11 : Subsystem  mask{bin_val1=48, bin_val2=49, bin_val3=50, bin_val4=51, bin_val5=52, bin_val6=53, bin_val7=54, bin_val8=55, bin_val9=56, bin_val10=57, bin_val11=58, bin_val12=59, bin_val13=60, bin_val14=61, bin_val15=62, bin_val16=63}
  Subsystem12 : Subsystem  mask{bin_val1=64, bin_val2=65, bin_val3=66, bin_val4=67, bin_val5=68, bin_val6=69, bin_val7=70, bin_val8=71, bin_val9=72, bin_val10=73, bin_val11=74, bin_val12=75, bin_val13=76, bin_val14=77, bin_val15=78, bin_val16=79}
  Subsystem13 : Subsystem  mask{bin_val1=80, bin_val2=81, bin_val3=82, bin_val4=83, bin_val5=84, bin_val6=85, bin_val7=86, bin_val8=87, bin_val9=88, bin_val10=89, bin_val11=90, bin_val12=91, bin_val13=92, bin_val14=93, bin_val15=94, bin_val16=95}
  Subsystem14 : Subsystem  mask{bin_val1=96, bin_val2=97, bin_val3=98, bin_val4=99, bin_val5=100, bin_val6=101, bin_val7=102, bin_val8=103, bin_val9=104, bin_val10=105, bin_val11=106, bin_val12=107, bin_val13=108, bin_val14=109, bin_val15=110, bin_val16=111}
  Subsystem15 : Subsystem  mask{bin_val1=112, bin_val2=113, bin_val3=114, bin_val4=115, bin_val5=116, bin_val6=117, bin_val7=118, bin_val8=119, bin_val9=120, bin_val10=121, bin_val11=122, bin_val12=123, bin_val13=124, bin_val14=125, bin_val15=126, bin_val16=127}
  Subsystem16 : Subsystem
  Subsystem2 : Subsystem  mask{bin_val1=-112, bin_val2=-111, bin_val3=-110, bin_val4=-109, bin_val5=-108, bin_val6=-107, bin_val7=-106, bin_val8=-105, bin_val9=-104, bin_val10=-103, bin_val11=-102, bin_val12=-101, bin_val13=-100, bin_val14=-99, bin_val15=-98, bin_val16=-97}
  Subsystem3 : Subsystem  mask{bin_val1=-80, bin_val2=-79, bin_val3=-78, bin_val4=-77, bin_val5=-76, bin_val6=-75, bin_val7=-74, bin_val8=-73, bin_val9=-72, bin_val10=-71, bin_val11=-70, bin_val12=-69, bin_val13=-68, bin_val14=-67, bin_val15=-66, bin_val16=-65}
  Subsystem4 : Subsystem  mask{bin_val1=-64, bin_val2=-63, bin_val3=-62, bin_val4=-61, bin_val5=-60, bin_val6=-59, bin_val7=-58, bin_val8=-57, bin_val9=-56, bin_val10=-55, bin_val11=-54, bin_val12=-53, bin_val13=-52, bin_val14=-51, bin_val15=-50, bin_val16=-49}
  Subsystem5 : Subsystem  mask{bin_val1=-48, bin_val2=-47, bin_val3=-46, bin_val4=-45, bin_val5=-44, bin_val6=-43, bin_val7=-42, bin_val8=-41, bin_val9=-40, bin_val10=-39, bin_val11=-38, bin_val12=-37, bin_val13=-36, bin_val14=-35, bin_val15=-34, bin_val16=-33}
  Subsystem6 : Subsystem  mask{bin_val1=-32, bin_val2=-31, bin_val3=-30, bin_val4=-29, bin_val5=-28, bin_val6=-27, bin_val7=-26, bin_val8=-25, bin_val9=-24, bin_val10=-23, bin_val11=-22, bin_val12=-21, bin_val13=-20, bin_val14=-19, bin_val15=-18, bin_val16=-17}
  Subsystem7 : Subsystem  mask{bin_val1=-16, bin_val2=-15, bin_val3=-14, bin_val4=-13, bin_val5=-12, bin_val6=-11, bin_val7=-10, bin_val8=-9, bin_val9=-8, bin_val10=-7, bin_val11=-6, bin_val12=-5, bin_val13=-4, bin_val14=-3, bin_val15=-2, bin_val16=-1}
  Subsystem8 : Subsystem  mask{bin_val1=0, bin_val2=1, bin_val3=2, bin_val4=3, bin_val5=4, bin_val6=5, bin_val7=6, bin_val8=7, bin_val9=8, bin_val10=9, bin_val11=10, bin_val12=11, bin_val13=12, bin_val14=13, bin_val15=14, bin_val16=15}
  Subsystem9 : Subsystem  mask{bin_val1=16, bin_val2=17, bin_val3=18, bin_val4=19, bin_val5=20, bin_val6=21, bin_val7=22, bin_val8=23, bin_val9=24, bin_val10=25, bin_val11=26, bin_val12=27, bin_val13=28, bin_val14=29, bin_val15=30, bin_val16=31}
  median_valid1 : Subsystem
  med_out : Outport
  med_valid : Outport  Port=2
  med_valid1 : Outport  Port=3
  wires:
  Register/1 -> median_valid1/1(reset_in)
  Mux/1 -> Mux/2, med_out/1, Scope2/2
  [med_loc] -> Subsystem/2(med_loc)
  [med_loc] -> Subsystem2/2(med_loc)
  [med_loc] -> Subsystem1/2(med_loc)
  [med_loc] -> Subsystem3/2(med_loc)
  [med_loc] -> Subsystem4/2(med_loc)
  [med_loc] -> Subsystem5/2(med_loc)
  [med_loc] -> Subsystem6/2(med_loc)
  [med_loc] -> Subsystem7/2(med_loc)
  [med_loc] -> Subsystem8/2(med_loc)
  [med_loc] -> Subsystem9/2(med_loc)
  [med_loc] -> Subsystem10/2(med_loc)
  [med_loc] -> Subsystem11/2(med_loc)
  [med_loc] -> Subsystem12/2(med_loc)
  [med_loc] -> Subsystem13/2(med_loc)
  [med_loc] -> Subsystem14/2(med_loc)
  [med_loc] -> Subsystem15/2(med_loc)
  [data_i] -> Subsystem/1(data_in)
  [data_i] -> Subsystem2/1(data_in)
  [data_i] -> Subsystem1/1(data_in)
  [data_i] -> Subsystem3/1(data_in)
  [data_i] -> Subsystem4/1(data_in)
  [data_i] -> Subsystem5/1(data_in)
  [data_i] -> Subsystem6/1(data_in)
  [data_i] -> Subsystem7/1(data_in)
  [data_i] -> Subsystem8/1(data_in)
  [data_i] -> Subsystem9/1(data_in)
  [data_i] -> Subsystem10/1(data_in)
  [data_i] -> Subsystem11/1(data_in)
  [data_i] -> Subsystem12/1(data_in)
  [data_i] -> Subsystem13/1(data_in)
  [data_i] -> Subsystem14/1(data_in)
  [data_i] -> Subsystem15/1(data_in)
  [acc_rst] -> Subsystem/3(acc_reset)
  [acc_rst] -> Subsystem2/3(acc_reset)
  [acc_rst] -> Subsystem1/3(acc_reset)
  [acc_rst] -> Subsystem3/3(acc_reset)
  [acc_rst] -> Subsystem4/3(acc_reset)
  [acc_rst] -> Subsystem5/3(acc_reset)
  [acc_rst] -> Subsystem6/3(acc_reset)
  [acc_rst] -> Subsystem7/3(acc_reset)
  [acc_rst] -> Subsystem8/3(acc_reset)
  [acc_rst] -> Subsystem9/3(acc_reset)
  [acc_rst] -> Subsystem10/3(acc_reset)
  [acc_rst] -> Subsystem11/3(acc_reset)
  [acc_rst] -> Subsystem12/3(acc_reset)
  [acc_rst] -> Subsystem13/3(acc_reset)
  [acc_rst] -> Subsystem14/3(acc_reset)
  [acc_rst] -> Subsystem15/3(acc_reset)
  data_in/1 -> [data_i]
  Constant/1 -> [med_loc]
  acc_reset/1 -> [acc_rst]
  Subsystem16/1(median_out) -> Mux/3, Scope3/1
  [acc_rst] -> Register/1, Delay/1
  Subsystem/1(priority_out1) -> [med_sel1]
  Subsystem/2(med_calc1) -> [med_in1]
  Subsystem2/1(priority_out1) -> [med_sel2]
  Subsystem2/2(med_calc1) -> [med_in2]
  Subsystem1/1(priority_out1) -> [med_sel3]
  Subsystem1/2(med_calc1) -> [med_in3]
  Subsystem3/1(priority_out1) -> [med_sel4]
  Subsystem3/2(med_calc1) -> [med_in4]
  Subsystem4/1(priority_out1) -> [med_sel5]
  Subsystem4/2(med_calc1) -> [med_in5]
  Subsystem5/1(priority_out1) -> [med_sel6]
  Subsystem5/2(med_calc1) -> [med_in6]
  Subsystem6/1(priority_out1) -> [med_sel7]
  Subsystem6/2(med_calc1) -> [med_in7]
  Subsystem7/1(priority_out1) -> [med_sel8], Scope1/1
  Subsystem7/2(med_calc1) -> [med_in8], Scope1/2
  Subsystem8/1(priority_out1) -> [med_sel9]
  Subsystem8/2(med_calc1) -> [med_in9]
  Subsystem9/1(priority_out1) -> [med_sel10]
  Subsystem9/2(med_calc1) -> [med_in10]
  Subsystem10/1(priority_out1) -> [med_sel11]
  Subsystem10/2(med_calc1) -> [med_in11]
  Subsystem11/1(priority_out1) -> [med_sel12]
  Subsystem11/2(med_calc1) -> [med_in12]
  Subsystem12/1(priority_out1) -> [med_sel13]
  Subsystem12/2(med_calc1) -> [med_in13]
  Subsystem13/1(priority_out1) -> [med_sel14]
  Subsystem13/2(med_calc1) -> [med_in14]
  Subsystem14/1(priority_out1) -> [med_sel15]
  Subsystem14/2(med_calc1) -> [med_in15]
  Subsystem15/1(priority_out1) -> [med_sel16]
  Subsystem15/2(med_calc1) -> [med_in16]
  [med_sel1] -> Subsystem16/1(med_sel1)
  [med_in1] -> Subsystem16/9(med_in1)
  [med_sel2] -> Subsystem16/2(med_sel2)
  [med_sel3] -> Subsystem16/3(med_sel3)
  [med_sel4] -> Subsystem16/4(med_sel4)
  [med_sel5] -> Subsystem16/5(med_sel5)
  [med_sel6] -> Subsystem16/6(med_sel6)
  [med_sel7] -> Subsystem16/7(med_sel7)
  [med_sel8] -> Subsystem16/8(med_sel8)
  [med_in2] -> Subsystem16/10(med_in2)
  [med_in3] -> Subsystem16/11(med_in3)
  [med_in4] -> Subsystem16/12(med_in4)
  [med_in5] -> Subsystem16/13(med_in5)
  [med_in6] -> Subsystem16/14(med_in6)
  [med_in7] -> Subsystem16/15(med_in7)
  [med_in8] -> Subsystem16/16(med_in8)
  [med_sel9] -> Subsystem16/17(med_sel9)
  [med_sel10] -> Subsystem16/18(med_sel10)
  [med_sel11] -> Subsystem16/19(med_sel11)
  [med_sel12] -> Subsystem16/20(med_sel12)
  [med_sel13] -> Subsystem16/21(med_sel13)
  [med_sel14] -> Subsystem16/22(med_sel14)
  [med_sel15] -> Subsystem16/23(med_sel15)
  [med_sel16] -> Subsystem16/24(med_sel16)
  [med_in9] -> Subsystem16/25(med_in9)
  [med_in10] -> Subsystem16/26(med_in10)
  [med_in11] -> Subsystem16/27(med_in11)
  [med_in12] -> Subsystem16/28(med_in12)
  [med_in13] -> Subsystem16/29(med_in13)
  [med_in14] -> Subsystem16/30(med_in14)
  [med_in15] -> Subsystem16/31(med_in15)
  [med_in16] -> Subsystem16/32(med_in16)
  Subsystem16/2(median_out_valid) -> Scope3/2
  Register2/1 -> Mux/1, Register1/1
  median_valid1/1(Out1) -> Register3/1
  Register3/1 -> Register2/1
  Delay/1 -> Expression/1
  Register1/1 -> Expression/2, med_valid1/1
  Expression/1 -> med_valid/1, Scope2/1

== system rfi_blk_v6_casper/rfi_blk/mad_comp/block_ram ==
  data : Inport
  Constant1 : xbsIndex_r4/Constant  arith_type=Boolean  const=1  n_bits=10  bin_pt=0  explicit_period=on  period=1  equ=P=C  opselect=C  inp2=PCIN>>17  opr=+  inp1=P  carry=CIN  dbl_ovrd=off  block_type=constant
  Constant2 : xbsIndex_r4/Constant  arith_type=Boolean  const=0  n_bits=10  bin_pt=0  explicit_period=on  period=1  equ=P=C  opselect=C  inp2=PCIN>>17  opr=+  inp1=P  carry=CIN  dbl_ovrd=off  block_type=constant
  Constant3 : xbsIndex_r4/Constant  arith_type=Signed (2's comp)  const=0  n_bits=8  bin_pt=0  explicit_period=on  period=1  equ=P=C  opselect=C  inp2=PCIN>>17  opr=+  inp1=P  carry=CIN  dbl_ovrd=off  block_type=constant
  Constant4 : xbsIndex_r4/Constant  arith_type=Boolean  const=1  n_bits=10  bin_pt=0  explicit_period=on  period=1  equ=P=C  opselect=C  inp2=PCIN>>17  opr=+  inp1=P  carry=CIN  dbl_ovrd=off  block_type=constant
  Counter2 : xbsIndex_r4/Counter  cnt_type=Free Running  cnt_to=2048  operation=Up  start_count=0  cnt_by_val=1  arith_type=Unsigned  n_bits=12  bin_pt=0  load_pin=off  rst=off  en=on  explicit_period=on  period=1  dbl_ovrd=off  use_behavioral_HDL=off  implementation=Fabric  use_rpm=off  block_type=counter
  Counter3 : xbsIndex_r4/Counter  cnt_type=Free Running  cnt_to=2048  operation=Up  start_count=0  cnt_by_val=1  arith_type=Unsigned  n_bits=12  bin_pt=0  load_pin=off  rst=off  en=off  explicit_period=on  period=1  dbl_ovrd=off  use_behavioral_HDL=off  implementation=Fabric  use_rpm=off  block_type=counter
  Delay1 : xbsIndex_r4/Delay  en=off  latency=1026  dbl_ovrd=off  reg_retiming=off  block_type=delay
  Dual Port RAM : xbsIndex_r4/Dual Port RAM  depth=2^12  initVector=sin(pi*(0:15)/16)  distributed_mem=Block RAM  init_a=0  init_b=0  rst_a=off  rst_b=off  en_a=off  en_b=off  latency=1  write_mode_A=Read After Write  write_mode_B=Read After Write  dbl_ovrd=off  optimize=Area  use_rpm=on  block_type=dpram
      settings: block_version=10.1.3
  Mux : xbsIndex_r4/Mux  inputs=2  en=off  latency=0  precision=Full  arith_type=Unsigned  n_bits=16  bin_pt=14  quantization=Truncate  overflow=Wrap  dbl_ovrd=off  block_type=mux
  Out1 : Outport
  Out2 : Outport  Port=2
  wires:
  Counter3/1 -> Dual Port RAM/1
  Constant1/1 -> Dual Port RAM/3
  data/1 -> Dual Port RAM/2, Dual Port RAM/5
  Constant2/1 -> Dual Port RAM/6
  Delay1/1 -> Counter2/1, Mux/1
  Counter2/1 -> Dual Port RAM/4
  Dual Port RAM/1 -> Out1/1
  Dual Port RAM/2 -> Mux/3
  Constant4/1 -> Delay1/1
  Constant3/1 -> Mux/2
  Mux/1 -> Out2/1

== system rfi_blk_v6_casper/rfi_blk/mad_comp/sub_abs_conv ==
  In1 : Inport
  In2 : Inport  Port=2
  AddSub1 : xbsIndex_r4/AddSub  mode=Subtraction  use_carryin=off  use_carryout=off  en=off  latency=1  precision=User Defined  arith_type="Signed  (2's comp)"  n_bits=8  bin_pt=0  quantization=Truncate  overflow=Wrap  dbl_ovrd=off  use_behavioral_HDL=off  hw_selection=Fabric  pipelined=off  use_rpm=on  block_type=addsub
      settings: block_version=10.1.3
  AddSub2 : xbsIndex_r4/AddSub  mode=Addition  use_carryin=off  use_carryout=off  en=off  latency=0  precision=Full  arith_type="Signed  (2's comp)"  n_bits=8  bin_pt=0  quantization=Truncate  overflow=Wrap  dbl_ovrd=off  use_behavioral_HDL=off  hw_selection=Fabric  pipelined=off  use_rpm=on  block_type=addsub
      settings: block_version=10.1.3
  AddSub3 : xbsIndex_r4/AddSub  mode=Subtraction  use_carryin=off  use_carryout=off  en=off  latency=0  precision=Full  arith_type="Signed  (2's comp)"  n_bits=8  bin_pt=0  quantization=Truncate  overflow=Wrap  dbl_ovrd=off  use_behavioral_HDL=off  hw_selection=Fabric  pipelined=off  use_rpm=on  block_type=addsub
      settings: block_version=10.1.3
  Constant1 : xbsIndex_r4/Constant  arith_type=Unsigned  const=255  n_bits=8  bin_pt=0  explicit_period=on  period=1  equ=P=C  opselect=C  inp2=PCIN>>17  opr=+  inp1=P  carry=CIN  dbl_ovrd=off  block_type=constant
  Constant4 : xbsIndex_r4/Constant  arith_type=Signed (2's comp)  const=0  n_bits=8  bin_pt=0  explicit_period=on  period=1  equ=P=C  opselect=C  inp2=PCIN>>17  opr=+  inp1=P  carry=CIN  dbl_ovrd=off  block_type=constant
  Constant5 : xbsIndex_r4/Constant  arith_type=Unsigned  const=255  n_bits=8  bin_pt=0  explicit_period=on  period=1  equ=P=C  opselect=C  inp2=PCIN>>17  opr=+  inp1=P  carry=CIN  dbl_ovrd=off  block_type=constant
  Delay : xbsIndex_r4/Delay  en=off  latency=1  dbl_ovrd=off  reg_retiming=off  block_type=delay
  Delay1 : xbsIndex_r4/Delay  Orientation=left  en=off  latency=1  dbl_ovrd=off  reg_retiming=off  block_type=delay
  Mux : xbsIndex_r4/Mux  inputs=2  en=off  latency=0  precision=Full  arith_type=Unsigned  n_bits=16  bin_pt=14  quantization=Truncate  overflow=Wrap  dbl_ovrd=off  block_type=mux
  Relational : xbsIndex_r4/Relational  mode=a<b  en=off  latency=1  dbl_ovrd=off  block_type=relational
      settings: block_version=10.1.3
  Out1 : Outport
  wires:
  AddSub1/1 -> AddSub2/1, Delay/1, Relational/1
  Constant4/1 -> Relational/2
  Relational/1 -> Mux/1
  AddSub2/1 -> AddSub3/2
  Constant1/1 -> AddSub2/2
  Constant5/1 -> AddSub3/1
  AddSub3/1 -> Delay1/1
  In1/1 -> AddSub1/1
  In2/1 -> AddSub1/2
  Mux/1 -> Out1/1
  Delay/1 -> Mux/2
  Delay1/1 -> Mux/3
